simulink model "adm_pcie_9h7_dts2x_dual_4x100g_dsp_8b"  (R2019a)
settings: Solver=VariableStepAuto  StartTime=0.0  StopTime=10.0  RelTol=1e-3
undefined symbols (not defined in the file or in its folder): datetime, fft_stages, posixtime
libraries: xbsIndex_r4 x21832, casper_library_misc x264, casper_library_sources x16, casper_library_delays x14, xps_library x11, casper_library_reorder x10, casper_library_scopes x8
(part 1 of 225)
settings Model: ModifiedComment=jackh -- Sat Mar 05 12:56:57 2022 -- Version 1.197  Creator=jackh  LastModifiedBy=jackh
settings Model: ModifiedHistory=|
  | jackh -- Sat Mar 05 12:56:57 2022 -- Version 1.197
  | 
  | jackh -- Thu Mar 03 13:10:05 2022 -- Version 1.196
  | 
  | set DTS to demux 2^3
  | 
  | jackh -- Thu Mar 03 13:08:43 2022 -- Version 1.195
  | 
  | jackh -- Thu Mar 03 13:08:01 2022 -- Version 1.194
  | 
  | jackh -- Tue Mar 01 20:34:16 2022 -- Version 1.193
  | 
  | jackh -- Tue Mar 01 17:19:43 2022 -- Version 1.192
  | 
  | jackh -- Tue Mar 01 17:10:04 2022 -- Version 1.191
  | 
  | jackh -- Tue Mar 01 16:55:54 2022 -- Version 1.190
  | 
  | jackh -- Tue Mar 01 16:45:57 2022 -- Version 1.189
  | 
  | jackh -- Tue Mar 01 15:57:56 2022 -- Version 1.188
  | 
  | jackh -- Tue Mar 01 15:49:45 2022 -- Version 1.187
  | 
  | jackh -- Tue Mar 01 15:22:14 2022 -- Version 1.186
  | 
  | jackh -- Mon Feb 28 13:26:19 2022 -- Version 1.185
  | 
  | jackh -- Mon Feb 28 13:08:19 2022 -- Version 1.184
  | 
  | jackh -- Sat Feb 26 14:41:54 2022 -- Version 1.183
  | 
  | jackh -- Fri Feb 25 18:46:11 2022 -- Version 1.182
  | 
  | jackh -- Fri Feb 25 18:45:03 2022 -- Version 1.181
  | 
  | jackh -- Thu Feb 24 15:51:36 2022 -- Version 1.180
  | 
  | update noisegen casting (just take MSBs)
  | 
  | jackh -- Thu Feb 24 15:08:58 2022 -- Version 1.179
  | 
  | v12
  | 
  | jackh -- Thu Feb 24 15:06:46 2022 -- Version 1.178
  | 
  | Move to 4x100G template
  | 
  | 
  | jackh -- Thu Feb 24 15:02:10 2022 -- Version 1.177
  | 
  | jackh -- Wed Feb 09 19:02:50 2022 -- Version 1.176
  | 
  | jackh -- Wed Feb 09 10:59:16 2022 -- Version 1.175
  | 
  | Try _again_ to fix timestamp issue.
  | This time the fix was actually simulated, and seems to work.
  | 
  | jackh -- Wed Feb 09 10:58:22 2022 -- Version 1.174
  | 
  | jackh -- Wed Feb 09 10:34:47 2022 -- Version 1.173
  | 
  | jackh -- Tue Feb 08 22:13:18 2022 -- Version 1.172
  | 
  | Change autocorr pipelining to fix timing errors
  | 
  | jackh -- Tue Feb 08 11:22:49 2022 -- Version 1.171
  | 
  | Try (again) to fix MCNT offset issue.
  | This time just propagate the time_step_bit lower MCNT counter
  | bits through to the header.
  | 
  | jackh -- Tue Feb 08 08:36:48 2022 -- Version 1.170
  | 
  | jackh -- Mon Feb 07 16:56:49 2022 -- Version 1.169
  | 
  | jackh -- Mon Feb 07 16:34:15 2022 -- Version 1.168
  | 
  | jackh -- Mon Feb 07 16:26:49 2022 -- Version 1.167
  | 
  | jackh -- Mon Feb 07 12:47:03 2022 -- Version 1.166
  | 
  | jackh -- Mon Feb 07 12:32:35 2022 -- Version 1.165
  | 
  | Add TT sync register, storing the time of the last sync
  | 
  | jackh -- Mon Feb 07 12:23:30 2022 -- Version 1.164
  | 
  | increment bugfix to 11.0.0.2
  | 
  | 
  | jackh -- Mon Feb 07 12:22:16 2022 -- Version 1.163
  | 
  | Propagate LSBs of sync time to packet headers
  | 
  | jackh -- Sat Feb 05 22:32:38 2022 -- Version 1.162
  | 
  | jackh -- Sat Feb 05 18:43:37 2022 -- Version 1.161
  | 
  | v11
  | More changes to reorder.
  | Process ADC data at 8 (not 12) bit resolution
  | Use URAM via black-box imports for coarse delay and big channel reorder
  | 
  | jackh -- Sat Feb 05 18:36:04 2022 -- Version 1.160
  | 
  | jackh -- Sat Feb 05 17:38:40 2022 -- Version 1.159
  | 
  | jackh -- Sat Feb 05 17:24:29 2022 -- Version 1.158
  | 
  | jackh -- Sat Feb 05 17:22:21 2022 -- Version 1.157
  | 
  | jackh -- Fri Feb 04 17:40:07 2022 -- Version 1.156
  | 
  | jackh -- Fri Feb 04 12:37:39 2022 -- Version 1.155
  | 
  | 64 times per packet
  | 
  | 
  | jackh -- Thu Feb 03 21:55:46 2022 -- Version 1.154
  | 
  | as compiled
  | 
  | 
  | jackh -- Thu Feb 03 14:45:04 2022 -- Version 1.153
  | 
  | jackh -- Thu Feb 03 14:28:02 2022 -- Version 1.152
  | 
  | jackh -- Thu Feb 03 13:59:18 2022 -- Version 1.151
  | 
  | jackh -- Thu Feb 03 11:15:21 2022 -- Version 1.150
  | 
  | jackh -- Wed Feb 02 16:47:47 2022 -- Version 1.149
  | 
  | jackh -- Wed Feb 02 16:40:11 2022 -- Version 1.148
  | 
  | Fix incorrect final transpose in reorder block
  | 
  | jackh -- Wed Feb 02 16:16:13 2022 -- Version 1.147
  | 
  | v8. Add channel reordering
  | 
  | Not actually necessary for 8-bit mode, but will
  | facilitate testing which is necessary for 3-bit mode
  | 
  | jackh -- Wed Feb 02 10:37:50 2022 -- Version 1.146
  | 
  | 7.0.1
  | 
  | Change coarse delay bram latency to 3 (hopefully will fix small timing fail)
  | 
  | jackh -- Wed Feb 02 10:34:03 2022 -- Version 1.145
  | 
  | jackh -- Tue Feb 01 14:52:02 2022 -- Version 1.144
  | 
  | Don't use ultraRAM for coarse delay (but we should!)
  | because it breaks sysgen. Sigh.
  | 
  | jackh -- Tue Feb 01 14:27:12 2022 -- Version 1.143
  | 
  | jackh -- Tue Feb 01 14:18:24 2022 -- Version 1.142
  | 
  | v7. Add noisegen / coarse delay
  | 
  | jackh -- Tue Feb 01 14:12:26 2022 -- Version 1.141
  | 
  | jackh -- Tue Feb 01 14:09:37 2022 -- Version 1.140
  | 
  | jackh -- Tue Feb 01 11:46:40 2022 -- Version 1.139
  | 
  | jackh -- Tue Feb 01 11:12:13 2022 -- Version 1.138
  | 
  | Add noise gen
  | 
  | jackh -- Tue Feb 01 11:01:17 2022 -- Version 1.137
  | 
  | v6.1 Harmonize input ordering convention by flipping
  | inputs so "input 0" is MSBs of data
  | 
  | jackh -- Tue Feb 01 10:38:57 2022 -- Version 1.136
  | 
  | jackh -- Mon Jan 31 13:11:04 2022 -- Version 1.135
  | 
  | jackh -- Mon Jan 31 12:33:38 2022 -- Version 1.134
  | 
  | jackh -- Mon Jan 31 12:25:15 2022 -- Version 1.133
  | 
  | jackh -- Fri Jan 28 17:22:49 2022 -- Version 1.132
  | 
  | jackh -- Fri Jan 28 17:16:19 2022 -- Version 1.131
  | 
  | jackh -- Fri Jan 28 17:06:25 2022 -- Version 1.130
  | 
  | v6.0 Add input bitstats / simulation mux
  | 
  | jackh -- Fri Jan 28 17:02:52 2022 -- Version 1.129
  | 
  | jackh -- Fri Jan 28 16:59:39 2022 -- Version 1.128
  | 
  | jackh -- Fri Jan 28 16:55:40 2022 -- Version 1.127
  | 
  | jackh -- Fri Jan 28 16:17:24 2022 -- Version 1.126
  | 
  | v5.0.2 Fix potential (but unliekly) issue with sync arm coinciding
  | with sync pulse
  | 
  | 
  | jackh -- Fri Jan 28 16:01:17 2022 -- Version 1.125
  | 
  | Attempt to fix DTS alignment unreliability (control signal CDC issue in mlib_devel)
  | 
  | jackh -- Fri Jan 28 12:51:42 2022 -- Version 1.124
  | 
  | as compiled
  | 
  | jackh -- Thu Jan 27 16:47:33 2022 -- Version 1.123
  | 
  | v5.0
  | 
  | Add sync gen logic
  | 
  | jackh -- Thu Jan 27 11:25:49 2022 -- Version 1.122
  | 
  | v4.1 Add index interval error counter
  | 
  | jackh -- Thu Jan 27 11:22:02 2022 -- Version 1.121
  | 
  | Make index 50ms -- add not-locked counter
  | 
  | 
  | jackh -- Thu Jan 27 10:32:00 2022 -- Version 1.120
  | 
  | Recompile using raw "index" pulse. Add index snapshots
  | 
  | jackh -- Wed Jan 26 15:06:47 2022 -- Version 1.119
  | 
  | jackh -- Wed Jan 26 13:46:28 2022 -- Version 1.118
  | 
  | jackh -- Wed Jan 26 13:04:56 2022 -- Version 1.117
  | 
  | jackh -- Tue Jan 25 18:32:15 2022 -- Version 1.116
  | 
  | Add index (10ms pulse?) count logic
  | 
  | jackh -- Tue Jan 25 18:31:36 2022 -- Version 1.115
  | 
  | jackh -- Tue Jan 25 11:48:12 2022 -- Version 1.114
  | 
  | jackh -- Tue Jan 25 11:41:44 2022 -- Version 1.113
  | 
  | jackh -- Tue Jan 25 11:38:37 2022 -- Version 1.112
  | 
  | Readd autocorr (needed behavioural complex mults)
  | 
  | jackh -- Mon Jan 24 20:49:54 2022 -- Version 1.111
  | 
  | comment out autocorr (causing compile fails)
  | 
  | jackh -- Mon Jan 24 20:10:36 2022 -- Version 1.110
  | 
  | jackh -- Mon Jan 24 19:58:33 2022 -- Version 1.109
  | 
  | jackh -- Mon Jan 24 19:53:28 2022 -- Version 1.108
  | 
  | autocorr floating point error fixes
  | 
  | 
  | jackh -- Mon Jan 24 19:51:34 2022 -- Version 1.107
  | 
  | jackh -- Mon Jan 24 18:28:31 2022 -- Version 1.106
  | 
  | jackh -- Mon Jan 24 18:27:12 2022 -- Version 1.105
  | 
  | Add autocorr (to pipeline 0)
  | 
  | jackh -- Mon Jan 24 18:15:24 2022 -- Version 1.104
  | 
  | Add EQTVG
  | 
  | jackh -- Mon Jan 24 13:19:54 2022 -- Version 1.103
settings DataIOCC: ReturnWorkspaceOutputs=off
settings DebuggingCC: PregeneratedLibrarySubsystemCodeDiagnostic=none  ArithmeticOperatorsInVariantConditions=warning
settings SFSimCC: SimUseLocalCustomCode=off
settings RTWCC: RTWUseLocalCustomCode=off

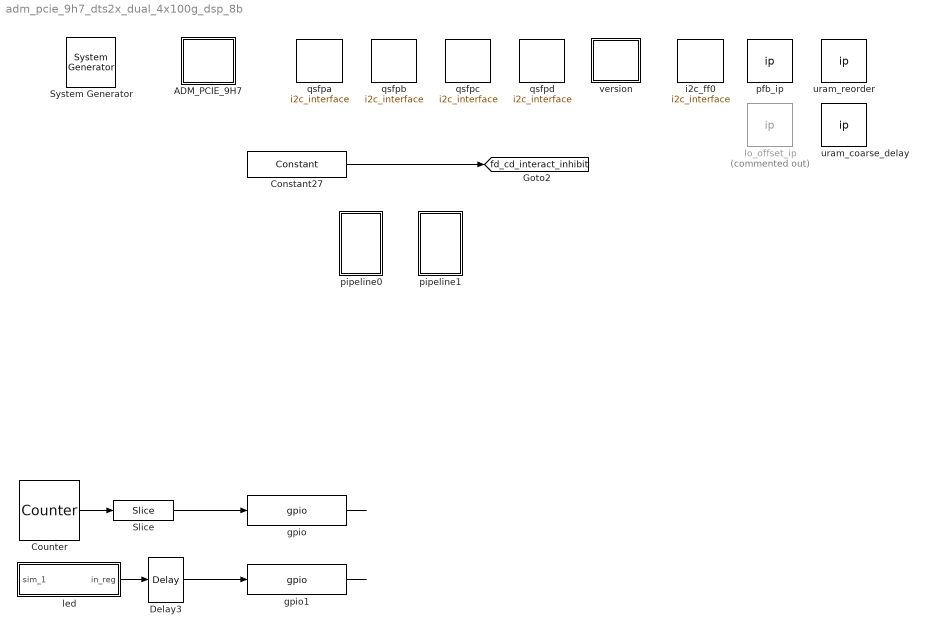
== system adm_pcie_9h7_dts2x_dual_4x100g_dsp_8b ==
  " System Generator" : xbsIndex_r4/ System Generator  Tag=genX  xilinxfamily=virtexuplusHBM  part=xcvu37p  speed=-2-e  package=fsvh2892  synthesis_tool=XST  clock_wrapper=Clock Enables  directory=/home/jackh/src/vla-dev/adm_pcie_9h7_dts2x_dual_4x100g_dsp_8b/sysgen  proj_type=Vivado  Synth_file=Vivado Synthesis Defaults  Impl_file=Vivado Implementation Defaults  testbench=off  simulink_period=1  sysclk_period=3.9062  dcm_input_clock_period=10  incr_netlist=off  trim_vbits=Everywhere in SubSystem  dbl_ovrd=According to Block Masks  core_generation=According to Block Masks  run_coregen=off  deprecated_control=off  eval_field=0  block_type=sysgen
  ADM_PCIE_9H7 : Subsystem  Tag=xps:xsg  mask(xsg core config){hw_sys=ADM_PCIE_9H7:xcvu37p, clk_src=dts_clk, clk_rate=256, use_microblaze=off, use_pr=on, pr_template='/home/jackh/src/vla-dev/pr_templates/adm_pcie_9h7_dts_dual_4x100g_pr_template-no-ip.xpr.zip', sample_period=1, synthesis_tool=XST}
      mask prompts: hw_sys "Hardware Platform"; clk_src "User IP Clock source"; clk_rate "User IP Clock Rate (MHz)"; use_microblaze "Use Microblaze controller?"; use_pr "Use PCIe Partial Reconfiguration"; sample_period "Sample Period"; synthesis_tool "Synthesis Tool:"
      mask help:
      | The XSG Core Config block is used to configure the System Generator design
      | for the casper_xps toolflow. Settings here are used to configure the
      | Xilinx System Generator block parameters automatically, and control toolflow
      | script  execution. It needs to be at the top level of all designs being compiled
      | with the casper_xps toolflow.
      mask init:
      | xps_xsg_adm_pcie_9h7_conf_mask;
      mask Help: eval('xlWeb([getenv(''MLIB_ROOT''), ''\doc\html\sys_xsg_core_config\sys_xsg_core_config.html''])')
      mask TypeOptions hw_sys: ADM_PCIE_9H7:xcvu37p
      mask TypeOptions clk_src: sys_clk | dts_clk
      mask TypeOptions synthesis_tool: Leonardo Spectrum | Synplify | Synplify Pro | XST
      mask Display: fprintf(['ADM\nPCIE_9H7']);
  Constant27 : xbsIndex_r4/Constant  const=1  gui_display_data_type=Boolean  arith_type=Boolean  n_bits=32  bin_pt=31  preci_type=Single  exp_width=8  frac_width=24  explicit_period=on  period=1  equ=P=C  opselect=C  inp2=PCIN>>17  opr=+  inp1=P  carry=CIN  dbl_ovrd=off  block_type=constant
  Counter : xbsIndex_r4/Counter  cnt_type=Free Running  cnt_to=Inf  operation=Up  start_count=0  cnt_by_val=1  arith_type=Unsigned  n_bits=27  bin_pt=0  load_pin=off  rst=off  en=off  explicit_period=on  period=1  use_behavioral_HDL=off  implementation=Fabric  dbl_ovrd=off  block_type=counter
  Delay3 : xbsIndex_r4/Delay  rst=off  infoeditControl=Selection of Reset will increase slice count due to use of real FFs and instead of SRLs  en=off  latency=1  reg_retiming=off  dbl_ovrd=off  block_type=delay
  Slice : xbsIndex_r4/Slice  nbits=2  boolean_output=off  mode=Upper Bit Location + Width  bit1=0  base1=MSB of Input  bit0=0  base0=LSB of Input  dbl_ovrd=off  block_type=slice
  gpio : xps_library/IO/gpio  Tag=xps:gpio  io_group_params_forwarded=off  io_group=ROACH:led  io_group_real=led  io_group_custom=0  io_dir=out  arith_type=Unsigned  bitwidth=2  bin_pt=0  bit_index=[0 1]  sample_period=1  use_single_ended=off  use_ddr=off  reg_iob=on  reg_clk_phase=0  termination=None
      settings: SourceProductName=CASPER XPS Blockset
  gpio1 : xps_library/IO/gpio  Tag=xps:gpio  io_group_params_forwarded=off  io_group=ROACH:led  io_group_real=led  io_group_custom=0  io_dir=out  arith_type=Boolean  bitwidth=1  bin_pt=0  bit_index=2  sample_period=1  use_single_ended=off  use_ddr=off  reg_iob=on  reg_clk_phase=0  termination=None
      settings: SourceProductName=CASPER XPS Blockset
  i2c_ff0 : xps_library/IO/i2c_interface  Tag=xps:i2c_interface  sda_gpio='gpio'  sda_gpio_index=8  scl_gpio='gpio'  scl_gpio_index=7
      settings: SourceProductName=CASPER XPS Blockset
  led : Subsystem  Tag=xps:sw_reg  AncestorBlock=xps_library/Memory/software_register  mask(swreg){io_dir=From Processor, io_delay=0, init_val=0, sample_period=1, names=reg, bitwidths=1, bin_pts=0, arith_types=2, sim_port=on, show_format=on}
      mask prompts: io_dir "I/O direction"; io_delay "I/O delay"; init_val "Initial Value"; sample_period "Sample period"; names "Bitfield names [msb...lsb]"; bitwidths "Bitfield widths"; bin_pts "Bitfield binary pts"; arith_types "Bitfield types, ufix=0, fix=1, bool=2"; sim_port "Provide sim input/output?"; show_format "Print format string?"
      mask help: A 32-bit software-accessible register. Can be divided into bitfields of varying widths and types using the fields in the block mask.
      mask init:
      | swreg_init(gcb)
      settings: AttributesFormatString=1input:b = 1 bits
      mask TypeOptions io_dir: To Processor | From Processor
      mask Callback io_dir:
      | mask_enables = get_param(gcb, 'MaskEnables');
      | mask_names = get_param(gcb, 'MaskNames');
      | if strcmp(get_param(gcb, 'io_dir'), 'To Processor'),
      |     mask_enables{ismember(mask_names, 'sample_period')} = 'off';
      | else
      |     mask_enables{ismember(mask_names, 'sample_period')} = 'on';
      | end
      | set_param(gcb, 'MaskEnables', mask_enables);
      mask DialogControl ParameterTabVar0 Prompt: Setup
  lo_offset_ip : xps_library/Utilities/ip  Tag=xps:ip  Commented=on  lib_path=[fileparts(which(bdroot)) '/dsp/mixer_dec_8x_8i_8o_core']  ip_name='mixer_dec_8x_8i_8o_core'
      settings: SourceProductName=CASPER XPS Blockset
  pfb_ip : xps_library/Utilities/ip  Tag=xps:ip  lib_path=[fileparts(which(bdroot)) '/dsp/pfb_cplx_2p_1024c_16i_18o_core']  ip_name='pfb_cplx_2p_1024c_16i_18o_core'
      settings: SourceProductName=CASPER XPS Blockset
  pipeline0 : Subsystem
  pipeline1 : Subsystem
  qsfpa : xps_library/IO/i2c_interface  Tag=xps:i2c_interface  sda_gpio='qsfp_sda'  sda_gpio_index=0  scl_gpio='qsfp_scl'  scl_gpio_index=0
      settings: SourceProductName=CASPER XPS Blockset
  qsfpb : xps_library/IO/i2c_interface  Tag=xps:i2c_interface  sda_gpio='qsfp_sda'  sda_gpio_index=1  scl_gpio='qsfp_scl'  scl_gpio_index=1
      settings: SourceProductName=CASPER XPS Blockset
  qsfpc : xps_library/IO/i2c_interface  Tag=xps:i2c_interface  sda_gpio='qsfp_sda'  sda_gpio_index=2  scl_gpio='qsfp_scl'  scl_gpio_index=2
      settings: SourceProductName=CASPER XPS Blockset
  qsfpd : xps_library/IO/i2c_interface  Tag=xps:i2c_interface  sda_gpio='qsfp_sda'  sda_gpio_index=3  scl_gpio='qsfp_scl'  scl_gpio_index=3
      settings: SourceProductName=CASPER XPS Blockset
  uram_coarse_delay : xps_library/Utilities/ip  Tag=xps:ip  lib_path=[fileparts(which(bdroot)) '/dsp/uram_dp_32kx64_latency3_core']  ip_name='uram_dp_32kx64_latency3_core'
      settings: SourceProductName=CASPER XPS Blockset
  uram_reorder : xps_library/Utilities/ip  Tag=xps:ip  lib_path=[fileparts(which(bdroot)) '/dsp/uram_8192x512_latency3_core']  ip_name='uram_8192x512_latency3_core'
      settings: SourceProductName=CASPER XPS Blockset
  version : Subsystem  mask{fw_type=2, ver_maj=12, ver_min=0, ver_rev=0, ver_bugfix=0}
      mask prompts: fw_type "Firmware Type"; ver_maj "Major version"; ver_min "Minor version"; ver_rev "Revision"; ver_bugfix "Bugfix"
  tag scope: fd_cd_interact_inhibit=global
  wires:
  gpio/1 -> ?
  Delay3/1 -> gpio1/1
  Counter/1 -> Slice/1
  gpio1/1 -> ?
  led/1(in_reg) -> Delay3/1
  Slice/1 -> gpio/1
  Constant27/1 -> [fd_cd_interact_inhibit]

== system adm_pcie_9h7_dts2x_dual_4x100g_dsp_8b/ADM_PCIE_9H7 ==

== system adm_pcie_9h7_dts2x_dual_4x100g_dsp_8b/led ==
  sim_1 : Inport
  adm_pcie_9h7_dts2x_dual_4x100g_dsp_8b_led_user_data_out : xbsIndex_r4/Gateway In  gui_display_data_type=Fixed-point  arith_type=Unsigned  n_bits=32  bin_pt=0  preci_type=Single  exp_width=8  frac_width=24  rows=1  columns=1  quantization="Round  (unbiased: +/- Inf)"  overflow=Saturate  period=1  UseAsADC=off  ADCChannel='1'  interface=None  automatic_assignment=on  address_offset=hex2dec('00')  intf_name=''  intf_description=''  default_value=0  timing_constraint=None  locs_specified=off  LOCs={}  io_standard={}  inherit_from_input=off  hdl_port=on  dbl_ovrd=off  block_type=gatewayin
  convert1_1 : DataTypeConversion  OutDataTypeStr=fixdt('ufix1_En0')  LockScale=on
  convert2_1 : DataTypeConversion  OutDataTypeStr=fixdt('ufix1_En0')  ConvertRealWorld=Stored Integer (SI)  LockScale=on
  gain_1 : Gain
  io_delay : xbsIndex_r4/Delay  rst=off  infoeditControl=Selection of Reset will increase slice count due to use of real FFs and instead of SRLs  en=off  latency=0  reg_retiming=on  dbl_ovrd=off  block_type=delay
  slice_reg : xbsIndex_r4/Slice  nbits=1  boolean_output=on  mode=Lower Bit Location + Width  bit1=0  base1=MSB of Input  bit0=0  base0=LSB of Input  dbl_ovrd=off  block_type=slice
  in_reg : Outport
  wires:
  adm_pcie_9h7_dts2x_dual_4x100g_dsp_8b_led_user_data_out/1 -> io_delay/1
  sim_1/1 -> convert1_1/1
  convert1_1/1 -> convert2_1/1
  convert2_1/1 -> gain_1/1
  gain_1/1 -> adm_pcie_9h7_dts2x_dual_4x100g_dsp_8b_led_user_data_out/1
  io_delay/1 -> slice_reg/1
  slice_reg/1 -> in_reg/1

== system adm_pcie_9h7_dts2x_dual_4x100g_dsp_8b/pipeline0 ==
  note: 4 inputs (2 IFs x dual pol)
      |
      | Each 8 parallel words x 12 bits
      | (96 bits per input)
      | (384 bits total)
  Constant : xbsIndex_r4/Constant  const=0  gui_display_data_type=Fixed-point  arith_type=Unsigned  n_bits=64  bin_pt=0  preci_type=Single  exp_width=8  frac_width=24  explicit_period=on  period=1  equ=P=C  opselect=C  inp2=PCIN>>17  opr=+  inp1=P  carry=CIN  dbl_ovrd=off  block_type=constant
  Constant1 : xbsIndex_r4/Constant  const=0  gui_display_data_type=Fixed-point  arith_type=Unsigned  n_bits=16  bin_pt=0  preci_type=Single  exp_width=8  frac_width=24  explicit_period=on  period=1  equ=P=C  opselect=C  inp2=PCIN>>17  opr=+  inp1=P  carry=CIN  dbl_ovrd=off  block_type=constant
  Constant2 : xbsIndex_r4/Constant  const=0  gui_display_data_type=Fixed-point  arith_type=Unsigned  n_bits=32  bin_pt=0  preci_type=Single  exp_width=8  frac_width=24  explicit_period=on  period=1  equ=P=C  opselect=C  inp2=PCIN>>17  opr=+  inp1=P  carry=CIN  dbl_ovrd=off  block_type=constant
  Constant3 : xbsIndex_r4/Constant  const=0  gui_display_data_type=Boolean  arith_type=Boolean  n_bits=32  bin_pt=0  preci_type=Single  exp_width=8  frac_width=24  explicit_period=on  period=1  equ=P=C  opselect=C  inp2=PCIN>>17  opr=+  inp1=P  carry=CIN  dbl_ovrd=off  block_type=constant
  Constant4 : xbsIndex_r4/Constant  const=0  gui_display_data_type=Boolean  arith_type=Boolean  n_bits=32  bin_pt=0  preci_type=Single  exp_width=8  frac_width=24  explicit_period=on  period=1  equ=P=C  opselect=C  inp2=PCIN>>17  opr=+  inp1=P  carry=CIN  dbl_ovrd=off  block_type=constant
  Constant5 : xbsIndex_r4/Constant  const=0  gui_display_data_type=Fixed-point  arith_type=Unsigned  n_bits=512  bin_pt=0  preci_type=Single  exp_width=8  frac_width=24  explicit_period=on  period=1  equ=P=C  opselect=C  inp2=PCIN>>17  opr=+  inp1=P  carry=CIN  dbl_ovrd=off  block_type=constant
  autocorr : Subsystem  mask{nchan_bits=9-2, npol_bits=0}
      mask prompts: nchan_bits "Number of *SERIAL* channels (2^?)"; npol_bits "Number of pols (2^?)"
  bus_convert1 : Subsystem  AncestorBlock=casper_library_bus/bus_convert  mask(bus_convert){n_bits_in=12*ones(4*8,1), bin_pt_in=11, cmplx=off, n_bits_out=8, bin_pt_out=7, quantization=0, overflow=0, csp_latency=0, of=off, misc=off}
      mask prompts: n_bits_in "input bit widths"; bin_pt_in "input binary points"; cmplx "input complex"; n_bits_out "output bit widths"; bin_pt_out "output binary points"; quantization "quantization strategy (Truncate=0, Round  (unbiased: +/- Inf)=1, Round (unbiased: Even Values)=2)"; overflow "overflow strategy (Wrap=0, Saturate=1, Flag as error=2)"; csp_latency "latency"; of "overflow indication"; misc "misc support"
      mask help: Convert components of busses
      mask init:
      | bus_convert_init(gcb, ...
      |     'n_bits_in', n_bits_in, ...
      |     'bin_pt_in', bin_pt_in, ...
      |     'cmplx', cmplx, ...
      |     'n_bits_out', n_bits_out, ...
      |     'bin_pt_out', bin_pt_out, ...
      |     'quantization', quantization, ...
      |     'overflow', overflow, ...
      |     'csp_latency', csp_latency, ...
      |     'misc', misc, ...
      |     'of', of);
  byte_flip : Subsystem  AncestorBlock=casper_library_flow_control/munge  mask(munge){divisions=64, div_size=8*ones(1,64), order=[63:-1:0], arith_type_out=Unsigned, bin_pt_out=0}
      mask prompts: divisions "Number of divisions"; div_size "Division size (bits)"; order "Division packing order"; arith_type_out "Output arithmetic type"; bin_pt_out "Output binary point"
      mask help: Splits an input bus into the specified number of divisions and then recombines them in the order specified (most significant division = 0,least = number divisions-1)
      mask init:
      | munge_init(gcb, ...
      |     'divisions', divisions, ...
      |     'div_size', div_size, ...
      |     'order', order, ...
      |     'arith_type_out', arith_type_out, ...
      |     'bin_pt_out', bin_pt_out);
      settings: AttributesFormatString=|
      | split:[8 8 8 8 8 8 8 8 8 8 8 8 8 8 8 8 8 8 8 8 8 8 8 8 8 8 8 8 8 8 8 8 8 8 8 8 8 8 8 8 8 8 8 8 8 8 8 8 8 8 8 8 8 8 8 8 8 8 8 8 8 8 8 8]
      | join:[63 62 61 60 59 58 57 56 55 54 53 52 51 50 49 48 47 46 45 44 43 42 41 40 39 38 37 36 35 34 33 32 31 30 29 28 27 26 25 24 23 22 21 20 19 18 17 16 15 14 13 12 11 10 9 8 7 6 5 4 3 2 1 0]
      | Unsigned [512,0]
      mask TypeOptions arith_type_out: Unsigned | Signed  (2's comp) | Floating-point
  delay : Subsystem
  dts : Subsystem
  eq : Subsystem
  eth0 : Subsystem
  eth1 : Subsystem
  input : Subsystem
  lo : Subsystem
  noise : Subsystem
  offset1 : Subsystem
  packetizer0 : Subsystem  mask{nchan_bits=9, npol_bits=(2+1)+6, nparallel_bits=5, step_bits=2, time_step_bits=6}
      mask prompts: nchan_bits "Number of channels (including unsent) (2^?)"; npol_bits "Number of pols*times (2^?)"; nparallel_bits "Parallel samples (2^?) Single-pol, single-chan, single-time samples per parallel word"; step_bits "Step (packet) granularity (2^?)"; time_step_bits "Time Steps per cycle (2^?). This is the amount the packet index is incremented by with each full sending cycle."
  pfb : Subsystem
  phase_rotate : Subsystem  mask{in_bits=18, n_chan_bits=9}
      mask prompts: in_bits "Data in bitwidth (per word)"; n_chan_bits "Number of frequency channels (2^?)"
  post_eq_tvg : Subsystem  mask{chan_bits=9, parallel_bits=2}
      mask prompts: chan_bits "Frequency Channels (2^?)"; parallel_bits "Parallel samples (2^?)"
  reorder : Subsystem
  sync : Subsystem  mask{sync_div_bits=30}
      mask prompts: sync_div_bits "Sync every 2^? FPGA clocks?"
  two_comp : Subsystem
  wires:
  offset1/1(dout) -> packetizer0/2(data_in)
  reorder/1(sync_out) -> packetizer0/1(sync_in)
  reorder/2(dout) -> offset1/1(din)
  packetizer0/1(data_out) -> byte_flip/1(din)
  packetizer0/3(eof) -> eth0/3(eof)
  packetizer0/4(dest_ip) -> eth0/4(dest_ip)
  packetizer0/5(dest_port) -> eth0/5(dest_port)
  packetizer0/6(byte_enable) -> eth0/6(byte_enable)
  byte_flip/1(dout) -> eth0/1(data)
  packetizer0/2(valid_out) -> eth0/2(vld)
  phase_rotate/1(sync_out) -> eq/1(sync)
  phase_rotate/2(dout) -> eq/2(din)
  pfb/1(sync_out) -> autocorr/1(sync), phase_rotate/1(sync)
  pfb/2(dout) -> autocorr/2(din), phase_rotate/2(din)
  [mrst] -> phase_rotate/3(mrst)
  eq/1(sync_out) -> post_eq_tvg/1(sync)
  eq/2(dout) -> post_eq_tvg/2(din)
  post_eq_tvg/1(sync_out) -> reorder/1(sync)
  post_eq_tvg/2(dout) -> reorder/2(din)
  [mrst] -> autocorr/3(rst)
  sync/3(ext_sync) -> [sync_trig]
  sync/2(tt_sync) -> [sync_time]
  sync/4(sync_out) -> [sync_gen]
  sync/1(arm_noise) -> [arm_noise]
  sync/5(mrst) -> [mrst]
  sync/6(adc_sync) -> [adc_sync]
  dts/1(sync) -> sync/1(sync_in)
  [mrst] -> packetizer0/3(mrst_in)
  [ver_maj] -> packetizer0/4(version)
  [sync_time] -> packetizer0/5(sync_time)
  [sync_gen] -> input/3(sync)
  dts/2(dout) -> two_comp/1(In1)
  [sync_gen] -> noise/1(sync_in)
  [arm_noise] -> noise/2(arm_noise)
  noise/1(noise) -> input/2(noise)
  bus_convert1/1(dout) -> input/1(adc)
  two_comp/1(Out1) -> bus_convert1/1(din)
  Constant/1 -> eth1/6(byte_enable)
  Constant1/1 -> eth1/5(dest_port)
  Constant2/1 -> eth1/4(dest_ip)
  Constant5/1 -> eth1/1(data)
  Constant4/1 -> eth1/2(vld)
  Constant3/1 -> eth1/3(eof)
  input/1(sync_out) -> delay/1(sync)
  input/2(dout) -> delay/2(din)
  [mrst] -> delay/3(mrst)
  [sync_time] -> delay/4(sync_time)
  delay/1(sync_out) -> lo/1(sync)
  delay/2(dout) -> lo/2(din)
  [mrst] -> lo/3(rst)
  lo/1(sync_out) -> pfb/1(sync)
  lo/2(dout) -> pfb/2(din)

== system adm_pcie_9h7_dts2x_dual_4x100g_dsp_8b/pipeline1 ==
  note: 4 inputs (2 IFs x dual pol)
      |
      | Each 8 parallel words x 12 bits
      | (96 bits per input)
      | (384 bits total)
  Constant : xbsIndex_r4/Constant  const=0  gui_display_data_type=Fixed-point  arith_type=Unsigned  n_bits=64  bin_pt=0  preci_type=Single  exp_width=8  frac_width=24  explicit_period=on  period=1  equ=P=C  opselect=C  inp2=PCIN>>17  opr=+  inp1=P  carry=CIN  dbl_ovrd=off  block_type=constant
  Constant1 : xbsIndex_r4/Constant  const=0  gui_display_data_type=Fixed-point  arith_type=Unsigned  n_bits=16  bin_pt=0  preci_type=Single  exp_width=8  frac_width=24  explicit_period=on  period=1  equ=P=C  opselect=C  inp2=PCIN>>17  opr=+  inp1=P  carry=CIN  dbl_ovrd=off  block_type=constant
  Constant2 : xbsIndex_r4/Constant  const=0  gui_display_data_type=Fixed-point  arith_type=Unsigned  n_bits=32  bin_pt=0  preci_type=Single  exp_width=8  frac_width=24  explicit_period=on  period=1  equ=P=C  opselect=C  inp2=PCIN>>17  opr=+  inp1=P  carry=CIN  dbl_ovrd=off  block_type=constant
  Constant3 : xbsIndex_r4/Constant  const=0  gui_display_data_type=Boolean  arith_type=Boolean  n_bits=32  bin_pt=0  preci_type=Single  exp_width=8  frac_width=24  explicit_period=on  period=1  equ=P=C  opselect=C  inp2=PCIN>>17  opr=+  inp1=P  carry=CIN  dbl_ovrd=off  block_type=constant
  Constant4 : xbsIndex_r4/Constant  const=0  gui_display_data_type=Boolean  arith_type=Boolean  n_bits=32  bin_pt=0  preci_type=Single  exp_width=8  frac_width=24  explicit_period=on  period=1  equ=P=C  opselect=C  inp2=PCIN>>17  opr=+  inp1=P  carry=CIN  dbl_ovrd=off  block_type=constant
  Constant5 : xbsIndex_r4/Constant  const=0  gui_display_data_type=Fixed-point  arith_type=Unsigned  n_bits=512  bin_pt=0  preci_type=Single  exp_width=8  frac_width=24  explicit_period=on  period=1  equ=P=C  opselect=C  inp2=PCIN>>17  opr=+  inp1=P  carry=CIN  dbl_ovrd=off  block_type=constant
  autocorr : Subsystem  mask{nchan_bits=9-2, npol_bits=0}
      mask prompts: nchan_bits "Number of *SERIAL* channels (2^?)"; npol_bits "Number of pols (2^?)"
  bus_convert1 : Subsystem  AncestorBlock=casper_library_bus/bus_convert  mask(bus_convert){n_bits_in=12*ones(4*8,1), bin_pt_in=11, cmplx=off, n_bits_out=8, bin_pt_out=7, quantization=0, overflow=0, csp_latency=0, of=off, misc=off}
      mask prompts: n_bits_in "input bit widths"; bin_pt_in "input binary points"; cmplx "input complex"; n_bits_out "output bit widths"; bin_pt_out "output binary points"; quantization "quantization strategy (Truncate=0, Round  (unbiased: +/- Inf)=1, Round (unbiased: Even Values)=2)"; overflow "overflow strategy (Wrap=0, Saturate=1, Flag as error=2)"; csp_latency "latency"; of "overflow indication"; misc "misc support"
      mask help: Convert components of busses
      mask init: same as adm_pcie_9h7_dts2x_dual_4x100g_dsp_8b/pipeline0/bus_convert1
  byte_flip : Subsystem  AncestorBlock=casper_library_flow_control/munge  mask(munge){divisions=64, div_size=8*ones(1,64), order=[63:-1:0], arith_type_out=Unsigned, bin_pt_out=0}
      mask prompts: divisions "Number of divisions"; div_size "Division size (bits)"; order "Division packing order"; arith_type_out "Output arithmetic type"; bin_pt_out "Output binary point"
      mask help: Splits an input bus into the specified number of divisions and then recombines them in the order specified (most significant division = 0,least = number divisions-1)
      mask init:
      | munge_init(gcb, ...
      |     'divisions', divisions, ...
      |     'div_size', div_size, ...
      |     'order', order, ...
      |     'arith_type_out', arith_type_out, ...
      |     'bin_pt_out', bin_pt_out);
      settings: AttributesFormatString=|
      | split:[8 8 8 8 8 8 8 8 8 8 8 8 8 8 8 8 8 8 8 8 8 8 8 8 8 8 8 8 8 8 8 8 8 8 8 8 8 8 8 8 8 8 8 8 8 8 8 8 8 8 8 8 8 8 8 8 8 8 8 8 8 8 8 8]
      | join:[63 62 61 60 59 58 57 56 55 54 53 52 51 50 49 48 47 46 45 44 43 42 41 40 39 38 37 36 35 34 33 32 31 30 29 28 27 26 25 24 23 22 21 20 19 18 17 16 15 14 13 12 11 10 9 8 7 6 5 4 3 2 1 0]
      | Unsigned [512,0]
      mask TypeOptions arith_type_out: Unsigned | Signed  (2's comp) | Floating-point
  delay : Subsystem
  dts : Subsystem
  eq : Subsystem
  eth0 : Subsystem
  eth1 : Subsystem
  input : Subsystem
  lo : Subsystem
  noise : Subsystem
  offset1 : Subsystem
  packetizer0 : Subsystem  mask{nchan_bits=9, npol_bits=(2+1)+6, nparallel_bits=5, step_bits=2, time_step_bits=6}
      mask prompts: nchan_bits "Number of channels (including unsent) (2^?)"; npol_bits "Number of pols*times (2^?)"; nparallel_bits "Parallel samples (2^?) Single-pol, single-chan, single-time samples per parallel word"; step_bits "Step (packet) granularity (2^?)"; time_step_bits "Time Steps per cycle (2^?). This is the amount the packet index is incremented by with each full sending cycle."
  pfb : Subsystem
  phase_rotate : Subsystem  mask{in_bits=18, n_chan_bits=9}
      mask prompts: in_bits "Data in bitwidth (per word)"; n_chan_bits "Number of frequency channels (2^?)"
  post_eq_tvg : Subsystem  mask{chan_bits=9, parallel_bits=2}
      mask prompts: chan_bits "Frequency Channels (2^?)"; parallel_bits "Parallel samples (2^?)"
  reorder : Subsystem
  sync : Subsystem  mask{sync_div_bits=30}
      mask prompts: sync_div_bits "Sync every 2^? FPGA clocks?"
  two_comp : Subsystem
  wires:
  two_comp/1(Out1) -> bus_convert1/1(din)
  noise/1(noise) -> input/2(noise)
  [arm_noise] -> noise/2(arm_noise)
  [sync_gen] -> noise/1(sync_in)
  dts/2(dout) -> two_comp/1(In1)
  [sync_gen] -> input/3(sync)
  [sync_time] -> packetizer0/5(sync_time)
  [ver_maj] -> packetizer0/4(version)
  [mrst] -> packetizer0/3(mrst_in)
  dts/1(sync) -> sync/1(sync_in)
  sync/6(adc_sync) -> [adc_sync]
  sync/5(mrst) -> [mrst]
  sync/1(arm_noise) -> [arm_noise]
  sync/4(sync_out) -> [sync_gen]
  sync/2(tt_sync) -> [sync_time]
  sync/3(ext_sync) -> [sync_trig]
  [mrst] -> autocorr/3(rst)
  post_eq_tvg/2(dout) -> reorder/2(din)
  post_eq_tvg/1(sync_out) -> reorder/1(sync)
  eq/2(dout) -> post_eq_tvg/2(din)
  eq/1(sync_out) -> post_eq_tvg/1(sync)
  [mrst] -> phase_rotate/3(mrst)
  pfb/2(dout) -> autocorr/2(din), phase_rotate/2(din)
  pfb/1(sync_out) -> autocorr/1(sync), phase_rotate/1(sync)
  phase_rotate/2(dout) -> eq/2(din)
  phase_rotate/1(sync_out) -> eq/1(sync)
  packetizer0/2(valid_out) -> eth0/2(vld)
  byte_flip/1(dout) -> eth0/1(data)
  packetizer0/6(byte_enable) -> eth0/6(byte_enable)
  packetizer0/5(dest_port) -> eth0/5(dest_port)
  packetizer0/4(dest_ip) -> eth0/4(dest_ip)
  packetizer0/3(eof) -> eth0/3(eof)
  packetizer0/1(data_out) -> byte_flip/1(din)
  reorder/2(dout) -> offset1/1(din)
  reorder/1(sync_out) -> packetizer0/1(sync_in)
  offset1/1(dout) -> packetizer0/2(data_in)
  bus_convert1/1(dout) -> input/1(adc)
  Constant1/1 -> eth1/5(dest_port)
  Constant/1 -> eth1/6(byte_enable)
  Constant3/1 -> eth1/3(eof)
  Constant5/1 -> eth1/1(data)
  Constant2/1 -> eth1/4(dest_ip)
  Constant4/1 -> eth1/2(vld)
  lo/1(sync_out) -> pfb/1(sync)
  lo/2(dout) -> pfb/2(din)
  input/1(sync_out) -> delay/1(sync)
  input/2(dout) -> delay/2(din)
  [mrst] -> delay/3(mrst)
  [sync_time] -> delay/4(sync_time)
  delay/1(sync_out) -> lo/1(sync)
  delay/2(dout) -> lo/2(din)
  [mrst] -> lo/3(rst)

== system adm_pcie_9h7_dts2x_dual_4x100g_dsp_8b/version ==
  Concat : xbsIndex_r4/Concat  num_inputs=4  dbl_ovrd=off  block_type=concat
  Constant : xbsIndex_r4/Constant  const=ver_maj  gui_display_data_type=Fixed-point  arith_type=Unsigned  n_bits=8  bin_pt=0  preci_type=Single  exp_width=8  frac_width=24  explicit_period=on  period=1  equ=P=C  opselect=C  inp2=PCIN>>17  opr=+  inp1=P  carry=CIN  dbl_ovrd=off  block_type=constant
  Constant1 : xbsIndex_r4/Constant  const=ver_min  gui_display_data_type=Fixed-point  arith_type=Unsigned  n_bits=8  bin_pt=0  preci_type=Single  exp_width=8  frac_width=24  explicit_period=on  period=1  equ=P=C  opselect=C  inp2=PCIN>>17  opr=+  inp1=P  carry=CIN  dbl_ovrd=off  block_type=constant
  Constant2 : xbsIndex_r4/Constant  const=ver_rev  gui_display_data_type=Fixed-point  arith_type=Unsigned  n_bits=8  bin_pt=0  preci_type=Single  exp_width=8  frac_width=24  explicit_period=on  period=1  equ=P=C  opselect=C  inp2=PCIN>>17  opr=+  inp1=P  carry=CIN  dbl_ovrd=off  block_type=constant
  Constant3 : xbsIndex_r4/Constant  const=ver_bugfix  gui_display_data_type=Fixed-point  arith_type=Unsigned  n_bits=8  bin_pt=0  preci_type=Single  exp_width=8  frac_width=24  explicit_period=on  period=1  equ=P=C  opselect=C  inp2=PCIN>>17  opr=+  inp1=P  carry=CIN  dbl_ovrd=off  block_type=constant
  Constant4 : xbsIndex_r4/Constant  const=fw_type  gui_display_data_type=Fixed-point  arith_type=Unsigned  n_bits=32  bin_pt=0  preci_type=Single  exp_width=8  frac_width=24  explicit_period=on  period=1  equ=P=C  opselect=C  inp2=PCIN>>17  opr=+  inp1=P  carry=CIN  dbl_ovrd=off  block_type=constant
  Terminator1 : Terminator
  Terminator2 : Terminator
  timestamp : Subsystem  Tag=xps:sw_reg  AncestorBlock=xps_library/Memory/software_register  mask(swreg){io_dir=To Processor, io_delay=0, init_val=0, sample_period=1, names=reg, bitwidths=32, bin_pts=0, arith_types=0, sim_port=on, show_format=off, desc_str=Unix timestamp, in integer seconds, when firmware was last compiled.}
      mask prompts: io_dir "I/O direction"; io_delay "I/O delay"; init_val "Initial Value"; sample_period "Sample period"; names "Bitfield names [msb...lsb]"; bitwidths "Bitfield widths"; bin_pts "Bitfield binary pts"; arith_types "Bitfield types, ufix=0, fix=1, bool=2"; sim_port "Provide sim input/output?"; show_format "Print format string?"; desc_str "Description"
      mask help: A 32-bit software-accessible register. Can be divided into bitfields of varying widths and types using the fields in the block mask.
      mask init:
      | swreg_init(gcb)
      settings: AttributesFormatString=1output
      mask TypeOptions io_dir: To Processor | From Processor
      mask Callback io_dir:
      | mask_enables = get_param(gcb, 'MaskEnables');
      | mask_names = get_param(gcb, 'MaskNames');
      | if strcmp(get_param(gcb, 'io_dir'), 'To Processor'),
      |     mask_enables{ismember(mask_names, 'sample_period')} = 'off';
      | else
      |     mask_enables{ismember(mask_names, 'sample_period')} = 'on';
      | end
      | set_param(gcb, 'MaskEnables', mask_enables);
      mask DialogControl ParameterTabVar0 Prompt: Setup
  type : Subsystem  Tag=xps:sw_reg  AncestorBlock=xps_library/Memory/software_register  mask(swreg){io_dir=To Processor, io_delay=0, init_val=0, sample_period=1, names=reg, bitwidths=32, bin_pts=0, arith_types=0, sim_port=on, show_format=off, desc_str=|}
      mask desc_str=|
      | Version register. Bits ``[31:24]``: major version. Bits
      | ``[23:16]``: minor version. Bits ``[15:8]``: revision.
      | Bits ``[7:0]``: buxfix.
      mask prompts: io_dir "I/O direction"; io_delay "I/O delay"; init_val "Initial Value"; sample_period "Sample period"; names "Bitfield names [msb...lsb]"; bitwidths "Bitfield widths"; bin_pts "Bitfield binary pts"; arith_types "Bitfield types, ufix=0, fix=1, bool=2"; sim_port "Provide sim input/output?"; show_format "Print format string?"; desc_str "Description"
      mask help: A 32-bit software-accessible register. Can be divided into bitfields of varying widths and types using the fields in the block mask.
      mask init:
      | swreg_init(gcb)
      settings: AttributesFormatString=1output
      mask TypeOptions io_dir: To Processor | From Processor
      mask Callback io_dir:
      | mask_enables = get_param(gcb, 'MaskEnables');
      | mask_names = get_param(gcb, 'MaskNames');
      | if strcmp(get_param(gcb, 'io_dir'), 'To Processor'),
      |     mask_enables{ismember(mask_names, 'sample_period')} = 'off';
      | else
      |     mask_enables{ismember(mask_names, 'sample_period')} = 'on';
      | end
      | set_param(gcb, 'MaskEnables', mask_enables);
      mask DialogControl ParameterTabVar0 Prompt: Setup
  unix_time : xbsIndex_r4/Constant  const=round(posixtime(datetime('now')))  gui_display_data_type=Fixed-point  arith_type=Unsigned  n_bits=32  bin_pt=0  preci_type=Single  exp_width=8  frac_width=24  explicit_period=on  period=1  equ=P=C  opselect=C  inp2=PCIN>>17  opr=+  inp1=P  carry=CIN  dbl_ovrd=off  block_type=constant
  version : Subsystem  Tag=xps:sw_reg  AncestorBlock=xps_library/Memory/software_register  mask(swreg){io_dir=To Processor, io_delay=0, init_val=0, sample_period=1, names=reg, bitwidths=32, bin_pts=0, arith_types=0, sim_port=on, show_format=off, desc_str=|}
      mask desc_str=|
      | Version register. Bits ``[31:24]``: major version. Bits
      | ``[23:16]``: minor version. Bits ``[15:8]``: revision.
      | Bits ``[7:0]``: buxfix.
      mask prompts: io_dir "I/O direction"; io_delay "I/O delay"; init_val "Initial Value"; sample_period "Sample period"; names "Bitfield names [msb...lsb]"; bitwidths "Bitfield widths"; bin_pts "Bitfield binary pts"; arith_types "Bitfield types, ufix=0, fix=1, bool=2"; sim_port "Provide sim input/output?"; show_format "Print format string?"; desc_str "Description"
      mask help: A 32-bit software-accessible register. Can be divided into bitfields of varying widths and types using the fields in the block mask.
      mask init:
      | swreg_init(gcb)
      settings: AttributesFormatString=1output
      mask TypeOptions io_dir: To Processor | From Processor
      mask Callback io_dir:
      | mask_enables = get_param(gcb, 'MaskEnables');
      | mask_names = get_param(gcb, 'MaskNames');
      | if strcmp(get_param(gcb, 'io_dir'), 'To Processor'),
      |     mask_enables{ismember(mask_names, 'sample_period')} = 'off';
      | else
      |     mask_enables{ismember(mask_names, 'sample_period')} = 'on';
      | end
      | set_param(gcb, 'MaskEnables', mask_enables);
      mask DialogControl ParameterTabVar0 Prompt: Setup
  tag scope: ver_maj=global
  wires:
  Constant1/1 -> Concat/2
  Concat/1 -> version/1(out_reg)
  Constant/1 -> [ver_maj], Concat/1
  version/1(sim_out_reg) -> Terminator1/1
  Constant2/1 -> Concat/3
  Constant3/1 -> Concat/4
  timestamp/1(sim_out_reg) -> Terminator2/1
  unix_time/1 -> timestamp/1(out_reg)
  Constant4/1 -> type/1(out_reg)

== system adm_pcie_9h7_dts2x_dual_4x100g_dsp_8b/pipeline0/autocorr ==
  note: acc_len in spectra
  sync : Inport
  din : Inport  Port=2
  rst : Inport  Port=3
  0 : Subsystem  mask{nchan_bits=nchan_bits, npol_bits=npol_bits}
      mask prompts: nchan_bits "Number of channels (2^?)"; npol_bits "Number of pols (2^?)"
  1 : Subsystem  mask{nchan_bits=nchan_bits, npol_bits=npol_bits}
      mask prompts: nchan_bits "Number of channels (2^?)"; npol_bits "Number of pols (2^?)"
  2 : Subsystem  mask{nchan_bits=nchan_bits, npol_bits=npol_bits}
      mask prompts: nchan_bits "Number of channels (2^?)"; npol_bits "Number of pols (2^?)"
  3 : Subsystem  mask{nchan_bits=nchan_bits, npol_bits=npol_bits}
      mask prompts: nchan_bits "Number of channels (2^?)"; npol_bits "Number of pols (2^?)"
  Constant6 : Constant  Value=1024
  Counter1 : xbsIndex_r4/Counter  cnt_type=Free Running  cnt_to=Inf  operation=Up  start_count=0  cnt_by_val=1  arith_type=Unsigned  n_bits=32  bin_pt=0  load_pin=off  rst=off  en=on  explicit_period=on  period=1  use_behavioral_HDL=on  implementation=Fabric  dbl_ovrd=off  block_type=counter
  Delay1 : xbsIndex_r4/Delay  rst=off  infoeditControl=Selection of Reset will increase slice count due to use of real FFs and instead of SRLs  en=off  latency=1  reg_retiming=off  dbl_ovrd=off  block_type=delay
  Delay2 : xbsIndex_r4/Delay  rst=off  infoeditControl=Selection of Reset will increase slice count due to use of real FFs and instead of SRLs  en=off  latency=1  reg_retiming=on  dbl_ovrd=off  block_type=delay
  Slice1 : xbsIndex_r4/Slice  nbits=32-nchan_bits-npol_bits  boolean_output=off  mode=Lower Bit Location + Width  bit1=0  base1=MSB of Input  bit0=0  base0=LSB of Input  dbl_ovrd=off  block_type=slice
  acc_cnt : Subsystem  Tag=xps:sw_reg  AncestorBlock=xps_library/Memory/software_register  mask(swreg){io_dir=To Processor, io_delay=0, init_val=0, sample_period=1, names=reg, bitwidths=32, bin_pts=0, arith_types=0, sim_port=on, show_format=on, desc_str=|}
      mask desc_str=|
      | 32-bit wrapping accumulation counter, which increments each
      | time a new accumulation is recorded to RAM.
      | There is no buffer locking when correlation data are
      | read, so this counter can be used to ensure that data
      | have not changed during a read.
      mask prompts: io_dir "I/O direction"; io_delay "I/O delay"; init_val "Initial Value"; sample_period "Sample period"; names "Bitfield names [msb...lsb]"; bitwidths "Bitfield widths"; bin_pts "Bitfield binary pts"; arith_types "Bitfield types, ufix=0, fix=1, bool=2"; sim_port "Provide sim input/output?"; show_format "Print format string?"; desc_str "Description"
      mask help: A 32-bit software-accessible register. Can be divided into bitfields of varying widths and types using the fields in the block mask.
      mask init:
      | swreg_init(gcb)
      settings: AttributesFormatString=1output:uf32.0 = 32 bits
      mask TypeOptions io_dir: To Processor | From Processor
      mask Callback io_dir:
      | mask_enables = get_param(gcb, 'MaskEnables');
      | mask_names = get_param(gcb, 'MaskNames');
      | if strcmp(get_param(gcb, 'io_dir'), 'To Processor'),
      |     mask_enables{ismember(mask_names, 'sample_period')} = 'off';
      | else
      |     mask_enables{ismember(mask_names, 'sample_period')} = 'on';
      | end
      | set_param(gcb, 'MaskEnables', mask_enables);
      mask DialogControl ParameterTabVar0 Prompt: Setup
  acc_len : Subsystem  Tag=xps:sw_reg  AncestorBlock=xps_library/Memory/software_register  mask(swreg){io_dir=From Processor, io_delay=0, init_val=0, sample_period=1, names=reg, bitwidths=32, bin_pts=0, arith_types=0, sim_port=on, show_format=on, desc_str=Accumulation length, in spectra.}
      mask prompts: io_dir "I/O direction"; io_delay "I/O delay"; init_val "Initial Value"; sample_period "Sample period"; names "Bitfield names [msb...lsb]"; bitwidths "Bitfield widths"; bin_pts "Bitfield binary pts"; arith_types "Bitfield types, ufix=0, fix=1, bool=2"; sim_port "Provide sim input/output?"; show_format "Print format string?"; desc_str "Description"
      mask help: A 32-bit software-accessible register. Can be divided into bitfields of varying widths and types using the fields in the block mask.
      mask init:
      | swreg_init(gcb)
      settings: AttributesFormatString=1input:uf32.0 = 32 bits
      mask TypeOptions io_dir: To Processor | From Processor
      mask Callback io_dir:
      | mask_enables = get_param(gcb, 'MaskEnables');
      | mask_names = get_param(gcb, 'MaskNames');
      | if strcmp(get_param(gcb, 'io_dir'), 'To Processor'),
      |     mask_enables{ismember(mask_names, 'sample_period')} = 'off';
      | else
      |     mask_enables{ismember(mask_names, 'sample_period')} = 'on';
      | end
      | set_param(gcb, 'MaskEnables', mask_enables);
      mask DialogControl ParameterTabVar0 Prompt: Setup
  bus_expand : Subsystem  AncestorBlock=casper_library_flow_control/bus_expand  mask(bus_expand){mode=divisions of equal size, outputNum=4, outputWidth=18*2*4, outputBinaryPt=0, outputArithmeticType=0, show_format=off, outputToWorkspace=off, variablePrefix=out, outputToModelAsWell=on}
      mask prompts: outputNum "Number of outputs:"; outputWidth "Output width:"; outputBinaryPt "Output binary point position:"; outputArithmeticType "Output arithmetic type (ufix=0, fix=1, bool=2):"; show_format "Print format string?"; outputToWorkspace "Output to workspace?"; variablePrefix "Variable name prefix:"; outputToModelAsWell "Output to model as well?"
      mask help:
      | Expands a 'bus' made using a bus_create or Xilinx
      | concat block.
      | The input will be divided into the specified number
      | of outputs with the specified arithmetic types.
      | Divisions are made from most significant bit.
      mask init:
      | bus_expand_init(gcb,...
      |     'mode', mode, ...
      |     'outputNum', outputNum, ...
      |     'outputWidth', outputWidth, ...
      |     'outputBinaryPt', outputBinaryPt, ...
      |     'outputArithmeticType', outputArithmeticType, ...
      |     'show_format', show_format, ...
      |     'outputToWorkspace', outputToWorkspace, ...
      |     'variablePrefix', variablePrefix, ...
      |     'outputToModelAsWell', outputToModelAsWell);
      settings: AttributesFormatString=4 outputs
      mask TypeOptions mode: divisions of equal size | divisions of arbitrary size
      mask Callback mode: bus_expand_callback();
      mask Callback outputWidth: bus_expand_callback();
      mask Callback outputToWorkspace: bus_expand_callback();
  trig : Subsystem  Tag=xps:sw_reg  AncestorBlock=xps_library/Memory/software_register  mask(swreg){io_dir=From Processor, io_delay=0, init_val=0, sample_period=1, names=reg, bitwidths=1, bin_pts=0, arith_types=2, sim_port=on, show_format=on, desc_str="Transition bit 0 from 0 to 1 to trigger a capture of the next valid accumulation"}
      mask prompts: io_dir "I/O direction"; io_delay "I/O delay"; init_val "Initial Value"; sample_period "Sample period"; names "Bitfield names [msb...lsb]"; bitwidths "Bitfield widths"; bin_pts "Bitfield binary pts"; arith_types "Bitfield types, ufix=0, fix=1, bool=2"; sim_port "Provide sim input/output?"; show_format "Print format string?"; desc_str "Description"
      mask help: A 32-bit software-accessible register. Can be divided into bitfields of varying widths and types using the fields in the block mask.
      mask init:
      | swreg_init(gcb)
      settings: AttributesFormatString=1input:b = 1 bits
      mask TypeOptions io_dir: To Processor | From Processor
      mask Callback io_dir:
      | mask_enables = get_param(gcb, 'MaskEnables');
      | mask_names = get_param(gcb, 'MaskNames');
      | if strcmp(get_param(gcb, 'io_dir'), 'To Processor'),
      |     mask_enables{ismember(mask_names, 'sample_period')} = 'off';
      | else
      |     mask_enables{ismember(mask_names, 'sample_period')} = 'on';
      | end
      | set_param(gcb, 'MaskEnables', mask_enables);
      mask DialogControl ParameterTabVar0 Prompt: Setup
  wires:
  din/1 -> bus_expand/1(bus_in)
  sync/1 -> [sync]
  rst/1 -> [rst]
  [sync] -> 0/1(sync)
  [rst] -> 0/3(rst)
  acc_len/1(in_reg) -> Slice1/1
  Constant6/1 -> acc_len/1(sim_1)
  Slice1/1 -> [acc_len]
  [new_acc] -> Delay1/1
  Delay2/1 -> acc_cnt/1(out_reg)
  Counter1/1 -> Delay2/1
  [acc_len] -> 0/4(acc_len)
  [acc_len] -> 1/4(acc_len)
  [rst] -> 1/3(rst)
  [sync] -> 1/1(sync)
  [sync] -> 2/1(sync)
  [rst] -> 2/3(rst)
  [acc_len] -> 2/4(acc_len)
  [acc_len] -> 3/4(acc_len)
  [rst] -> 3/3(rst)
  [sync] -> 3/1(sync)
  bus_expand/1(msb_out4) -> [input0]
  bus_expand/2(out3) -> [input1]
  bus_expand/3(out2) -> [input2]
  bus_expand/4(lsb_out1) -> [input3]
  [input1] -> 1/2(din)
  [input0] -> 0/2(din)
  trig/1(in_reg) -> [trig]
  [trig] -> 0/5(trig)
  0/1(new_acc) -> [new_acc]
  [trig] -> 1/5(trig)
  [trig] -> 2/5(trig)
  [trig] -> 3/5(trig)
  [input2] -> 2/2(din)
  [input3] -> 3/2(din)
  Delay1/1 -> Counter1/1
